simulink model "wlan_phy_rx_pmd"  (Simulink 7.8)
settings: Solver=VariableStepDiscrete  SolverMode=SingleTasking  StartTime=0.0  StopTime=rx_sim_time  RelTol=1e-3
PostLoadFcn:
  | wlan_phy_rx_init
InitFcn:
  | wlan_phy_rx_init
StartFcn:
  | wlan_phy_rx_init
values from neighbouring files (symbols this model uses):
  barker_seq20 = 0.95 * circshift(barker_seq20, [0 4]) ./ max(abs(barker_seq20))    [wlan_phy_rx_init.m]
  bit_scrambler_lfsr_bytes = sum(bit_scrambler_lfsr_bytes .* repmat(2.^[0:7], 127, 1),2)    [wlan_phy_rx_init.m]
  deinterleave_ROM = [deinterleave_ROM interleave_11n_64QAM zeros(1, 512-length(interleave_11n_64QAM))]    [wlan_phy_rx_init.m]
  longCorr_coef_i = [3*real(longCorr_coef)]    [wlan_phy_rx_init.m]
  longCorr_coef_q = [3*imag(longCorr_coef)]    [wlan_phy_rx_init.m]
  MAX_CP_LEN = 32    [wlan_phy_rx_init.m]
  MAX_NCBPS = NCBPS_11n_64QAM    (= 312)    [wlan_phy_rx_init.m]
  MAX_NUM_BYTES = 2^16-1    (= 65535)    [wlan_phy_rx_init.m]
  MAX_NUM_SC = 64    [wlan_phy_rx_init.m]
  MAX_NUM_SYMS = 600    [wlan_phy_rx_init.m]
  NCBPS_11a_BPSK = 48    [wlan_phy_rx_init.m]
  NDBPS_11a_BPSK12 = 24    [wlan_phy_rx_init.m]
  PHY_SIGNAL_MIN_LEN = 14    [wlan_phy_rx_init.m]
  preFFT_sampBuff_numSamps = 4*MAX_NUM_SC    (= 256)    [wlan_phy_rx_init.m]
  rx_sim_sig_samp_time = 8    [wlan_phy_rx_init.m]
  scr_ind_rev = zeros(1,128)    [wlan_phy_rx_init.m]
undefined symbols (not defined in the file or in its folder): gcbh, mcs_rom_11ag, mcs_rom_11n, xlBlockLoadCallback, xlGetNormalizedPeriod, xlipgetpartsetting
libraries: xbsIndex_r4 x3862, simulink x1, xbsEDKLib_r4 x1
(part 13 of 23)

== system wlan_phy_rx_pmd/DSSS Rx/Demod/Sync/SFD Search ==
  Bit : Inport
  Bit Valid : Inport  Port=2
  8 Shift Reg : Subsystem
  8 Shift Reg1 : Subsystem
  Concat1 : xbsIndex_r4/Concat  num_inputs=2  dbl_ovrd=off  block_type=concat
  Constant2 : xbsIndex_r4/Constant  const=hex2dec('F3A0')  gui_display_data_type=Fixed-point  arith_type=Unsigned  n_bits=16  bin_pt=0  preci_type=Single  exp_width=8  frac_width=24  explicit_period=off  period=1  equ=P=C  opselect=C  inp2=PCIN>>17  opr=+  inp1=P  carry=CIN  dbl_ovrd=off  block_type=constant
  Logical : xbsIndex_r4/Logical  logical_function=AND  inputs=2  en=off  latency=0  precision=Full  arith_type=Unsigned  n_bits=8  bin_pt=2  align_bp=on  dbl_ovrd=off  block_type=logical
      settings: xl_area=[0, 0, 0, 0, 0, 0, 0]  block_version=VER_STRING_GOES_HERE
  Posedge : Subsystem
  Relational1 : xbsIndex_r4/Relational  mode=a=b  en=off  latency=0  dbl_ovrd=off  block_type=relational
  Found : Outport
  wires:
  Logical/1 -> Posedge/1(D)
  Bit/1 -> 8 Shift Reg/1(D)
  Bit Valid/1 -> 8 Shift Reg/2(En), Logical/2, 8 Shift Reg1/2(En)
  [DSSS_RX_RESET] -> 8 Shift Reg/3(Rst), 8 Shift Reg1/3(Rst)
  8 Shift Reg/1(Q) -> 8 Shift Reg1/1(D)
  8 Shift Reg/2(8b) -> Concat1/1
  8 Shift Reg1/2(8b) -> Concat1/2
  Concat1/1 -> Relational1/2
  Constant2/1 -> Relational1/1
  Relational1/1 -> Logical/1
  Posedge/1(Q) -> Found/1

== system wlan_phy_rx_pmd/DSSS Rx/Sym Sync/Sym Sync Timeout/Posedge ==
  D : Inport
  Delay : xbsIndex_r4/Delay  rst=off  infoeditControl=Selection of Reset will increase slice count due to use of real FFs and instead of SRLs  en=off  latency=1  dbl_ovrd=off  reg_retiming=off  block_type=delay
      settings: infoedit=Hardware notes: A delay line is a chain, each link of which is an SRL16 followed by a flip-flop. If register retiming is enabled, the delay line is a chain of flip-flops.  xl_area=[1 1 0 0 0 0 0]  block_version=VER_STRING_GOES_HERE
  Inverter : xbsIndex_r4/Inverter  en=off  latency=0  dbl_ovrd=off  block_type=inv
      settings: xl_area=[0 0 0 0 0 0 0]  block_version=VER_STRING_GOES_HERE
  Logical1 : xbsIndex_r4/Logical  logical_function=AND  inputs=2  en=off  latency=0  precision=Full  arith_type=Unsigned  n_bits=8  bin_pt=2  align_bp=on  dbl_ovrd=off  block_type=logical
      settings: xl_area=[1 0 0 1 0 0 0]  block_version=VER_STRING_GOES_HERE
  Q : Outport
  wires:
  D/1 -> Logical1/2, Delay/1
  Logical1/1 -> Q/1
  Inverter/1 -> Logical1/1
  Delay/1 -> Inverter/1

== system wlan_phy_rx_pmd/DSSS Rx/Sym Sync/Sym Sync Timeout/S-R Latch2 ==
  S : Inport
  R : Inport  Port=2
  Constant1 : xbsIndex_r4/Constant  const=1  gui_display_data_type=Boolean  arith_type=Boolean  n_bits=1  bin_pt=0  preci_type=Single  exp_width=8  frac_width=24  explicit_period=off  period=1  equ=P=C  opselect=C  inp2=PCIN>>17  opr=+  inp1=P  carry=CIN  dbl_ovrd=off  block_type=constant
      settings: block_version=VER_STRING_GOES_HERE
  Convert1 : xbsIndex_r4/Convert  gui_display_data_type=Boolean  arith_type=Boolean  n_bits=1  bin_pt=0  float_type=Single  exp_bits=8  fraction_bits=24  quantization=Truncate  overflow=Wrap  en=off  latency=0  dbl_ovrd=off  pipeline=off  block_type=convert
  Convert2 : xbsIndex_r4/Convert  gui_display_data_type=Boolean  arith_type=Boolean  n_bits=1  bin_pt=0  float_type=Single  exp_bits=8  fraction_bits=24  quantization=Truncate  overflow=Wrap  en=off  latency=0  dbl_ovrd=off  pipeline=off  block_type=convert
  Register2 : xbsIndex_r4/Register  init=0  rst=on  en=on  dbl_ovrd=off  block_type=register
      settings: xl_area=[1 1 0 0 0 0 0]  block_version=VER_STRING_GOES_HERE
  Q : Outport
  wires:
  Convert2/1 -> Register2/3
  Convert1/1 -> Register2/2
  Constant1/1 -> Register2/1
  R/1 -> Convert1/1
  Register2/1 -> Q/1
  S/1 -> Convert2/1

== system wlan_phy_rx_pmd/Detect & Decode/De-Interleave & De-Puncture/Read Addr Gen/Ctrl ==
  note: This accumulator counts the number of actual bits that have been read
      | from the de-interleaver RAM. For 1/2 rate code this is easy (2 bits per read).
      | For the punctured code rates some read cycles represent 0, 1 or 2 bits. This is
      | important as the de-interleaver RAM only contains received bits and not the
      | zero-confidence values for de-punctured bits. De-puncturing is achieved by
      | not incrementing the read address and forcing the corresponding LLR
      | output to be zero (the value which means 'coin flip' for the decoder)
  mod_sel : Inport
  rate_sel : Inport  Port=2
  Reset : Inport  Port=3
  En : Inport  Port=4
  SIGNAL sym : Inport  Port=5
  Bit Index Calc : Subsystem
  Data Bits Count : Subsystem
  De Int ctrl : Scope  NumInputPorts=8
      settings: TimeRange=18000  YMin=0~0~0~0~0~0~-1~0  YMax=1~2~1~1~100~800~1~1  SaveName=ScopeData38
  Gateway Out{1..8} (8 alike) : xbsIndex_r4/Gateway Out  inherit_from_input=off  hdl_port=off  timing_constraint=None  locs_specified=off  LOCs={}  UseAsDAC=off  DACChannel='1'  block_type=gatewayout
  Mux1 : xbsIndex_r4/Mux  inputs=4  en=off  latency=0  precision=Full  arith_type=Unsigned  n_bits=16  bin_pt=14  quantization=Truncate  overflow=Wrap  dbl_ovrd=off  block_type=mux
  Mux3 : xbsIndex_r4/Mux  inputs=4  en=off  latency=0  precision=Full  arith_type=Unsigned  n_bits=16  bin_pt=14  quantization=Truncate  overflow=Wrap  dbl_ovrd=off  block_type=mux
  Mux4 : xbsIndex_r4/Mux  inputs=4  en=off  latency=0  precision=Full  arith_type=Unsigned  n_bits=16  bin_pt=14  quantization=Truncate  overflow=Wrap  dbl_ovrd=off  block_type=mux
  Rate 1/2 : Subsystem
  Rate 2/3 : Subsystem
  Rate 3/4 : Subsystem
  Rate 5/6 : Subsystem
  Ind A : Outport
  Ind B : Outport  Port=2
  Skip A : Outport  Port=3
  Skip B : Outport  Port=4
  Vout : Outport  Port=5
  Done : Outport  Port=6
  wires:
  Mux3/1 -> Skip A/1
  Rate 1/2/1(Ind Inc) -> [R12_INC_A]
  Rate 1/2/2(Skip A) -> [R12_SKIP_A]
  Rate 1/2/3(Skip B) -> [R12_SKIP_B]
  Rate 2/3/1(Ind Inc) -> [R23_INC_A]
  Rate 2/3/2(Skip A) -> [R23_SKIP_A]
  Rate 2/3/3(Skip B) -> [R23_SKIP_B]
  Rate 3/4/1(Ind Inc) -> [R34_INC_A]
  Rate 3/4/2(Skip A) -> [R34_SKIP_A]
  Rate 3/4/3(Skip B) -> [R34_SKIP_B]
  [R12_SKIP_A] -> Mux3/2
  [R23_SKIP_A] -> Mux3/3
  [R34_SKIP_A] -> Mux3/4
  Mux1/1 -> Skip B/1
  [R12_SKIP_B] -> Mux1/2
  [R23_SKIP_B] -> Mux1/3
  [R34_SKIP_B] -> Mux1/4
  [R12_INC_A] -> Mux4/2
  [R23_INC_A] -> Mux4/3
  [R34_INC_A] -> Mux4/4
  Mux4/1 -> Bit Index Calc/3(Inc)
  Gateway Out2/1 -> De Int ctrl/1  "Mod"
  Gateway Out1/1 -> De Int ctrl/2  "Rate"
  Gateway Out3/1 -> De Int ctrl/3  "Rst"
  Gateway Out4/1 -> De Int ctrl/4  "En"
  Gateway Out5/1 -> De Int ctrl/5  "A"
  Gateway Out7/1 -> De Int ctrl/8  "Vout"
  Data Bits Count/1(Done) -> Done/1, Gateway Out8/1
  Gateway Out8/1 -> De Int ctrl/7  "Done"
  mod_sel/1 -> Gateway Out2/1, Bit Index Calc/1(Mod)
  rate_sel/1 -> Mux4/1, Mux3/1, Mux1/1, Gateway Out1/1
  Reset/1 -> Gateway Out3/1, Bit Index Calc/4(Rst), Rate 2/3/1(Rst), Rate 3/4/1(Rst), Rate 5/6/1(Rst), Rate 1/2/1(Rst)
  En/1 -> Vout/1, Gateway Out7/1, Bit Index Calc/5(En), Rate 2/3/2(En), Rate 3/4/2(En), Rate 5/6/2(En), Rate 1/2/2(En), Gateway Out4/1, Data Bits Count/1(En)
  SIGNAL sym/1 -> Data Bits Count/2(SIGNAL sym), Bit Index Calc/2(SIGNAL Sym)
  Bit Index Calc/1(Ind A) -> Ind A/1, Gateway Out5/1
  Bit Index Calc/2(Ind B) -> Ind B/1, Gateway Out6/1  "B"
  Gateway Out6/1 -> De Int ctrl/6  "B"
  [R56_INC_A] -> Mux4/5
  [R56_SKIP_A] -> Mux3/5
  Rate 5/6/1(Ind Inc) -> [R56_INC_A]
  Rate 5/6/2(Skip A) -> [R56_SKIP_A]
  Rate 5/6/3(Skip B) -> [R56_SKIP_B]
  [R56_SKIP_B] -> Mux1/5

== system wlan_phy_rx_pmd/Detect & Decode/De-Interleave & De-Puncture/Read Addr Gen/De-Interleave DP-ROM ==
  Ind A : Inport
  Ind B : Inport  Port=2
  Constant8 : xbsIndex_r4/Constant  const=0  gui_display_data_type=Fixed-point  arith_type=Unsigned  n_bits=ceil(log2(MAX_NCBPS))  bin_pt=0  preci_type=Single  exp_width=8  frac_width=24  explicit_period=off  period=1  equ=P=C  opselect=C  inp2=PCIN>>17  opr=+  inp1=P  carry=CIN  dbl_ovrd=off  block_type=constant
  Constant9 : xbsIndex_r4/Constant  const=0  gui_display_data_type=Boolean  arith_type=Boolean  n_bits=2  bin_pt=0  preci_type=Single  exp_width=8  frac_width=24  explicit_period=off  period=1  equ=P=C  opselect=C  inp2=PCIN>>17  opr=+  inp1=P  carry=CIN  dbl_ovrd=off  block_type=constant
  De-Interleave ROM : xbsIndex_r4/Dual Port RAM  depth=length(deinterleave_ROM)  initVector=deinterleave_ROM  distributed_mem=Block RAM  init_a=0  init_b=0  rst_a=off  rst_b=off  en_a=off  en_b=off  latency=1  write_mode_A=Read After Write  write_mode_B=Read After Write  dbl_ovrd=off  optimize=Area  block_type=dpram
  Addr A : Outport
  Addr B : Outport  Port=2
  wires:
  Constant8/1 -> De-Interleave ROM/2, De-Interleave ROM/5
  Constant9/1 -> De-Interleave ROM/3, De-Interleave ROM/6
  Ind A/1 -> De-Interleave ROM/1
  De-Interleave ROM/1 -> Addr A/1
  Ind B/1 -> De-Interleave ROM/4
  De-Interleave ROM/2 -> Addr B/1

== system wlan_phy_rx_pmd/Detect & Decode/De-Interleave & De-Puncture/Read Addr Gen/S-R Latch1 ==
  S : Inport
  R : Inport  Port=2
  Constant1 : xbsIndex_r4/Constant  const=1  gui_display_data_type=Fixed-point  arith_type=Boolean  n_bits=1  bin_pt=0  preci_type=Single  exp_width=8  frac_width=24  explicit_period=off  period=1  equ=P=C  opselect=C  inp2=PCIN>>17  opr=+  inp1=P  carry=CIN  dbl_ovrd=off  block_type=constant
      settings: block_version=VER_STRING_GOES_HERE
  Convert1 : xbsIndex_r4/Convert  gui_display_data_type=Boolean  arith_type=Boolean  n_bits=1  bin_pt=0  float_type=Single  exp_bits=8  fraction_bits=24  quantization=Truncate  overflow=Wrap  en=off  latency=0  dbl_ovrd=off  pipeline=off  block_type=convert
  Convert2 : xbsIndex_r4/Convert  gui_display_data_type=Boolean  arith_type=Boolean  n_bits=1  bin_pt=0  float_type=Single  exp_bits=8  fraction_bits=24  quantization=Truncate  overflow=Wrap  en=off  latency=0  dbl_ovrd=off  pipeline=off  block_type=convert
  Register2 : xbsIndex_r4/Register  init=0  rst=on  en=on  dbl_ovrd=off  block_type=register
      settings: xl_area=[1 1 0 0 0 0 0]  block_version=VER_STRING_GOES_HERE
  Q : Outport
  wires:
  Convert2/1 -> Register2/3
  Convert1/1 -> Register2/2
  Constant1/1 -> Register2/1
  R/1 -> Convert1/1
  Register2/1 -> Q/1
  S/1 -> Convert2/1

== system wlan_phy_rx_pmd/Detect & Decode/Decode/Byte Count/S-R Latch1 ==
  S : Inport
  R : Inport  Port=2
  Constant1 : xbsIndex_r4/Constant  const=1  gui_display_data_type=Fixed-point  arith_type=Boolean  n_bits=1  bin_pt=0  preci_type=Single  exp_width=8  frac_width=24  explicit_period=off  period=1  equ=P=C  opselect=C  inp2=PCIN>>17  opr=+  inp1=P  carry=CIN  dbl_ovrd=off  block_type=constant
      settings: block_version=VER_STRING_GOES_HERE
  Convert1 : xbsIndex_r4/Convert  gui_display_data_type=Boolean  arith_type=Boolean  n_bits=1  bin_pt=0  float_type=Single  exp_bits=8  fraction_bits=24  quantization=Truncate  overflow=Wrap  en=off  latency=0  dbl_ovrd=off  pipeline=off  block_type=convert
  Convert2 : xbsIndex_r4/Convert  gui_display_data_type=Boolean  arith_type=Boolean  n_bits=1  bin_pt=0  float_type=Single  exp_bits=8  fraction_bits=24  quantization=Truncate  overflow=Wrap  en=off  latency=0  dbl_ovrd=off  pipeline=off  block_type=convert
  Register2 : xbsIndex_r4/Register  init=0  rst=on  en=on  dbl_ovrd=off  block_type=register
      settings: xl_area=[1 1 0 0 0 0 0]  block_version=VER_STRING_GOES_HERE
  Q : Outport
  wires:
  Convert2/1 -> Register2/3
  Convert1/1 -> Register2/2
  Constant1/1 -> Register2/1
  R/1 -> Convert1/1
  Register2/1 -> Q/1
  S/1 -> Convert2/1

== system wlan_phy_rx_pmd/Detect & Decode/Decode/Control/Early Trace Ctrl ==
  note: 22: (NUM_SIGNAL_BYTES * 8) - 2
  note: 70: ((NUM_SIGNAL_BYTES + NUM_HT_SIGNAL_BYTES)* 8) - 2
  note: early_trace inputs require pulse coincident
      | with last vin for early bits, staying asserted
      | until at least first decoder vout.
  Byte Ind : Inport
  dec_vout : Inport  Port=2
  reset : Inport  Port=3
  Constant1 : xbsIndex_r4/Constant  const=9*8 - 2  gui_display_data_type=Fixed-point  arith_type=Unsigned  n_bits=7  bin_pt=0  preci_type=Single  exp_width=8  frac_width=24  explicit_period=off  period=1  equ=P=C  opselect=C  inp2=PCIN>>17  opr=+  inp1=P  carry=CIN  dbl_ovrd=off  block_type=constant
  Constant3 : xbsIndex_r4/Constant  const=3*8 - 2  gui_display_data_type=Fixed-point  arith_type=Unsigned  n_bits=5  bin_pt=0  preci_type=Single  exp_width=8  frac_width=24  explicit_period=off  period=1  equ=P=C  opselect=C  inp2=PCIN>>17  opr=+  inp1=P  carry=CIN  dbl_ovrd=off  block_type=constant
  Convert : xbsIndex_r4/Convert  gui_display_data_type=Boolean  arith_type=Boolean  n_bits=16  bin_pt=14  float_type=Single  exp_bits=8  fraction_bits=24  quantization=Truncate  overflow=Wrap  en=off  latency=0  dbl_ovrd=off  pipeline=off  block_type=convert
  Convert1 : xbsIndex_r4/Convert  gui_display_data_type=Boolean  arith_type=Boolean  n_bits=16  bin_pt=14  float_type=Single  exp_bits=8  fraction_bits=24  quantization=Truncate  overflow=Wrap  en=off  latency=0  dbl_ovrd=off  pipeline=off  block_type=convert
  Logical1 : xbsIndex_r4/Logical  logical_function=AND  inputs=2  en=off  latency=0  precision=Full  arith_type=Unsigned  n_bits=16  bin_pt=0  align_bp=on  dbl_ovrd=off  block_type=logical
  Logical2 : xbsIndex_r4/Logical  logical_function=OR  inputs=2  en=off  latency=0  precision=Full  arith_type=Unsigned  n_bits=16  bin_pt=0  align_bp=on  dbl_ovrd=off  block_type=logical
  Relational1 : xbsIndex_r4/Relational  mode=a=b  en=off  latency=0  dbl_ovrd=off  block_type=relational
  Relational3 : xbsIndex_r4/Relational  mode=a=b  en=off  latency=0  dbl_ovrd=off  block_type=relational
  S-R Latch1 : Subsystem
  S-R Latch3 : Subsystem
  posedge : Subsystem
  posedge1 : Subsystem
  Early Trace 1 : Outport
  Early Trace 2 : Outport  Port=2
  wires:
  Constant3/1 -> Relational3/1
  Byte Ind/1 -> Relational3/2, Relational1/1
  Relational3/1 -> posedge/1(D)
  Constant1/1 -> Relational1/2
  Relational1/1 -> Logical1/1
  [RX_PHY_MODE_11N_AC] -> Logical1/2
  Logical1/1 -> posedge1/1(D)
  posedge/1(Q) -> S-R Latch1/1(S)
  posedge1/1(Q) -> S-R Latch3/1(S)
  dec_vout/1 -> Convert/1
  reset/1 -> Convert1/1
  Logical2/1 -> S-R Latch1/2(R), S-R Latch3/2(R)
  S-R Latch1/1(Q) -> Early Trace 1/1
  S-R Latch3/1(Q) -> Early Trace 2/1
  Convert/1 -> Logical2/1
  Convert1/1 -> Logical2/2

== system wlan_phy_rx_pmd/Detect & Decode/Decode/Control/negedge ==
  D : Inport
  Delay : xbsIndex_r4/Delay  rst=off  infoeditControl=Selection of Reset will increase slice count due to use of real FFs and instead of SRLs  en=off  latency=1  dbl_ovrd=off  reg_retiming=off  block_type=delay
      settings: infoedit=Hardware notes: A delay line is a chain, each link of which is an SRL16 followed by a flip-flop. If register retiming is enabled, the delay line is a chain of flip-flops.  xl_area=[1 1 0 0 0 0 0]  block_version=VER_STRING_GOES_HERE
  Inverter : xbsIndex_r4/Inverter  en=off  latency=0  dbl_ovrd=off  block_type=inv
      settings: xl_area=[0 0 0 0 0 0 0]  block_version=VER_STRING_GOES_HERE
  Logical1 : xbsIndex_r4/Logical  logical_function=AND  inputs=2  en=off  latency=0  precision=Full  arith_type=Unsigned  n_bits=8  bin_pt=2  align_bp=on  dbl_ovrd=off  block_type=logical
      settings: xl_area=[1 0 0 1 0 0 0]  block_version=VER_STRING_GOES_HERE
  Q : Outport
  wires:
  D/1 -> Delay/1, Inverter/1
  Logical1/1 -> Q/1
  Delay/1 -> Logical1/1
  Inverter/1 -> Logical1/2

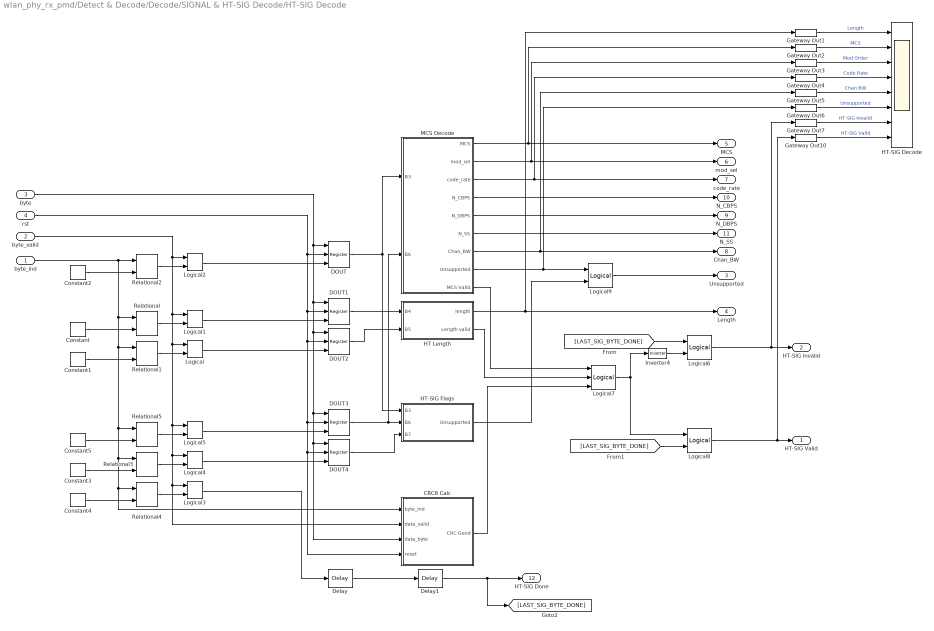
== system wlan_phy_rx_pmd/Detect & Decode/Decode/SIGNAL & HT-SIG Decode/HT-SIG Decode ==
  byte_ind : Inport
  byte_valid : Inport  Port=2
  byte : Inport  Port=3
  rst : Inport  Port=4
  CRC8 Calc : Subsystem
  Constant : xbsIndex_r4/Constant  const=4  gui_display_data_type=Fixed-point  arith_type=Unsigned  n_bits=3  bin_pt=0  preci_type=Single  exp_width=8  frac_width=24  explicit_period=off  period=1  equ=P=C  opselect=C  inp2=PCIN>>17  opr=+  inp1=P  carry=CIN  dbl_ovrd=off  block_type=constant
  Constant1 : xbsIndex_r4/Constant  const=5  gui_display_data_type=Fixed-point  arith_type=Unsigned  n_bits=3  bin_pt=0  preci_type=Single  exp_width=8  frac_width=24  explicit_period=off  period=1  equ=P=C  opselect=C  inp2=PCIN>>17  opr=+  inp1=P  carry=CIN  dbl_ovrd=off  block_type=constant
  Constant2 : xbsIndex_r4/Constant  const=3  gui_display_data_type=Fixed-point  arith_type=Unsigned  n_bits=2  bin_pt=0  preci_type=Single  exp_width=8  frac_width=24  explicit_period=off  period=1  equ=P=C  opselect=C  inp2=PCIN>>17  opr=+  inp1=P  carry=CIN  dbl_ovrd=off  block_type=constant
  Constant3 : xbsIndex_r4/Constant  const=7  gui_display_data_type=Fixed-point  arith_type=Unsigned  n_bits=3  bin_pt=0  preci_type=Single  exp_width=8  frac_width=24  explicit_period=off  period=1  equ=P=C  opselect=C  inp2=PCIN>>17  opr=+  inp1=P  carry=CIN  dbl_ovrd=off  block_type=constant
  Constant4 : xbsIndex_r4/Constant  const=8  gui_display_data_type=Fixed-point  arith_type=Unsigned  n_bits=4  bin_pt=0  preci_type=Single  exp_width=8  frac_width=24  explicit_period=off  period=1  equ=P=C  opselect=C  inp2=PCIN>>17  opr=+  inp1=P  carry=CIN  dbl_ovrd=off  block_type=constant
  Constant5 : xbsIndex_r4/Constant  const=6  gui_display_data_type=Fixed-point  arith_type=Unsigned  n_bits=3  bin_pt=0  preci_type=Single  exp_width=8  frac_width=24  explicit_period=off  period=1  equ=P=C  opselect=C  inp2=PCIN>>17  opr=+  inp1=P  carry=CIN  dbl_ovrd=off  block_type=constant
  DOUT : xbsIndex_r4/Register  init=0  rst=on  en=on  dbl_ovrd=off  block_type=register
  DOUT1 : xbsIndex_r4/Register  init=100  rst=on  en=on  dbl_ovrd=off  block_type=register
  DOUT2 : xbsIndex_r4/Register  init=0  rst=on  en=on  dbl_ovrd=off  block_type=register
  DOUT3 : xbsIndex_r4/Register  init=hex2dec('03')  rst=on  en=on  dbl_ovrd=off  block_type=register
  DOUT4 : xbsIndex_r4/Register  init=hex2dec('E0')  rst=on  en=on  dbl_ovrd=off  block_type=register
  Delay : xbsIndex_r4/Delay  rst=off  infoeditControl=Selection of Reset will increase slice count due to use of real FFs and instead of SRLs  en=off  latency=1  dbl_ovrd=off  reg_retiming=off  block_type=delay
  Delay1 : xbsIndex_r4/Delay  rst=off  infoeditControl=Selection of Reset will increase slice count due to use of real FFs and instead of SRLs  en=off  latency=1  dbl_ovrd=off  reg_retiming=off  block_type=delay
  Gateway Out{1..7,10} (8 alike) : xbsIndex_r4/Gateway Out  inherit_from_input=off  hdl_port=off  timing_constraint=None  locs_specified=off  LOCs={}  UseAsDAC=off  DACChannel='1'  block_type=gatewayout
  HT Length : Subsystem
  HT-SIG Decode : Scope  NumInputPorts=8
      settings: TimeRange=7000  YMin=0~0~0~0~100~-1~-1~-1  YMax=1~1~150~1~300~1~1~1  SaveName=ScopeData45
  HT-SIG Flags : Subsystem
  Inverter4 : xbsIndex_r4/Inverter  en=off  latency=0  dbl_ovrd=off  block_type=inv
  Logical{,1..6,8} (8 alike) : xbsIndex_r4/Logical  logical_function=AND  inputs=2  en=off  latency=0  precision=Full  arith_type=Unsigned  n_bits=16  bin_pt=0  align_bp=on  dbl_ovrd=off  block_type=logical
  Logical7 : xbsIndex_r4/Logical  logical_function=AND  inputs=3  en=off  latency=0  precision=Full  arith_type=Unsigned  n_bits=16  bin_pt=0  align_bp=on  dbl_ovrd=off  block_type=logical
  Logical9 : xbsIndex_r4/Logical  logical_function=OR  inputs=2  en=off  latency=0  precision=Full  arith_type=Unsigned  n_bits=16  bin_pt=0  align_bp=on  dbl_ovrd=off  block_type=logical
  MCS Decode : Subsystem
  Relational{,1..5} (6 alike) : xbsIndex_r4/Relational  mode=a=b  en=off  latency=0  dbl_ovrd=off  block_type=relational
  HT-SIG Valid : Outport
  HT-SIG Invalid : Outport  Port=2
  Unsupported : Outport  Port=3
  Length : Outport  Port=4
  MCS : Outport  Port=5
  mod_sel : Outport  Port=6
  code_rate : Outport  Port=7
  Chan_BW : Outport  Port=8
  N_DBPS : Outport  Port=9
  N_CBPS : Outport  Port=10
  N_SS : Outport  Port=11
  HT-SIG Done : Outport  Port=12
  wires:
  rst/1 -> DOUT1/2, DOUT2/2, DOUT3/2, DOUT4/2, CRC8 Calc/4(reset), DOUT/2
  Logical1/1 -> DOUT1/3
  Logical2/1 -> DOUT/3
  Logical/1 -> DOUT2/3
  Relational/1 -> Logical1/2
  Relational2/1 -> Logical2/2
  Relational1/1 -> Logical/2
  Constant2/1 -> Relational2/2
  Constant1/1 -> Relational1/2
  Constant/1 -> Relational/2
  byte/1 -> DOUT1/1, DOUT2/1, DOUT4/1, CRC8 Calc/3(data_byte), DOUT3/1, DOUT/1
  byte_ind/1 -> Relational/1, Relational1/1, Relational3/1, Relational4/1, CRC8 Calc/1(byte_ind), Relational5/1, Relational2/1
  byte_valid/1 -> Logical1/1, Logical/1, Logical4/1, Logical3/1, CRC8 Calc/2(data_valid), Logical5/1, Logical2/1
  Logical4/1 -> DOUT4/3
  Logical5/1 -> DOUT3/3
  Logical3/1 -> Delay/1
  Relational3/1 -> Logical4/2
  Relational5/1 -> Logical5/2
  Relational4/1 -> Logical3/2
  Constant5/1 -> Relational5/2
  Constant4/1 -> Relational4/2
  Constant3/1 -> Relational3/2
  DOUT/1 -> HT-SIG Flags/1(B3), MCS Decode/1(B3)
  DOUT1/1 -> HT Length/1(B4)
  DOUT2/1 -> HT Length/2(B5)
  DOUT3/1 -> HT-SIG Flags/2(B6), MCS Decode/2(B6)
  Gateway Out1/1 -> HT-SIG Decode/1  "Length"
  Gateway Out10/1 -> HT-SIG Decode/8  "HT-SIG Valid"
  Gateway Out2/1 -> HT-SIG Decode/2  "MCS"
  Gateway Out3/1 -> HT-SIG Decode/3  "Mod Order"
  Gateway Out4/1 -> HT-SIG Decode/4  "Code Rate"
  MCS Decode/1(MCS) -> MCS/1, Gateway Out2/1
  MCS Decode/2(mod_sel) -> mod_sel/1, Gateway Out3/1
  MCS Decode/3(code_rate) -> code_rate/1, Gateway Out4/1
  MCS Decode/4(N_CBPS) -> N_CBPS/1
  MCS Decode/5(N_DBPS) -> N_DBPS/1
  MCS Decode/6(N_SS) -> N_SS/1
  MCS Decode/7(Chan_BW) -> Chan_BW/1, Gateway Out5/1
  HT Length/1(length) -> Length/1, Gateway Out1/1
  [LAST_SIG_BYTE_DONE] -> Logical6/1
  Logical7/1 -> Inverter4/1, Logical8/1
  [LAST_SIG_BYTE_DONE] -> Logical8/2
  Logical6/1 -> HT-SIG Invalid/1, Gateway Out7/1
  Logical8/1 -> HT-SIG Valid/1, Gateway Out10/1
  MCS Decode/9(MCS Valid) -> Logical7/1
  HT Length/2(Length valid) -> Logical7/2
  CRC8 Calc/1(CRC Good) -> Logical7/3
  Delay/1 -> Delay1/1
  HT-SIG Flags/1(Unsupported) -> Logical9/2
  MCS Decode/8(Unsupported) -> Gateway Out6/1, Logical9/1
  Gateway Out5/1 -> HT-SIG Decode/5  "Chan BW"
  Gateway Out6/1 -> HT-SIG Decode/6  "Unsupported"
  Delay1/1 -> [LAST_SIG_BYTE_DONE], HT-SIG Done/1
  DOUT4/1 -> HT-SIG Flags/3(B7)
  Logical9/1 -> Unsupported/1
  Gateway Out7/1 -> HT-SIG Decode/7  "HT-SIG Invalid"
  Inverter4/1 -> Logical6/2

== system wlan_phy_rx_pmd/Detect & Decode/Decode/SIGNAL & HT-SIG Decode/S-R Latch1 ==
  S : Inport
  R : Inport  Port=2
  Constant1 : xbsIndex_r4/Constant  const=1  gui_display_data_type=Fixed-point  arith_type=Boolean  n_bits=1  bin_pt=0  preci_type=Single  exp_width=8  frac_width=24  explicit_period=off  period=1  equ=P=C  opselect=C  inp2=PCIN>>17  opr=+  inp1=P  carry=CIN  dbl_ovrd=off  block_type=constant
      settings: block_version=VER_STRING_GOES_HERE
  Convert1 : xbsIndex_r4/Convert  gui_display_data_type=Boolean  arith_type=Boolean  n_bits=1  bin_pt=0  float_type=Single  exp_bits=8  fraction_bits=24  quantization=Truncate  overflow=Wrap  en=off  latency=0  dbl_ovrd=off  pipeline=off  block_type=convert
  Convert2 : xbsIndex_r4/Convert  gui_display_data_type=Boolean  arith_type=Boolean  n_bits=1  bin_pt=0  float_type=Single  exp_bits=8  fraction_bits=24  quantization=Truncate  overflow=Wrap  en=off  latency=0  dbl_ovrd=off  pipeline=off  block_type=convert
  Register2 : xbsIndex_r4/Register  init=0  rst=on  en=on  dbl_ovrd=off  block_type=register
      settings: xl_area=[1 1 0 0 0 0 0]  block_version=VER_STRING_GOES_HERE
  Q : Outport
  wires:
  Convert2/1 -> Register2/3
  Convert1/1 -> Register2/2
  Constant1/1 -> Register2/1
  R/1 -> Convert1/1
  Register2/1 -> Q/1
  S/1 -> Convert2/1

== system wlan_phy_rx_pmd/Detect & Decode/Decode/SIGNAL & HT-SIG Decode/SIGNAL Decode ==
  note: Init values of these regs are important
      | Decoder black box requires valid params at start,
      | so these regs are initialzied such that:
      | mod_order = 0 (BSPK)
      | code_rate = 0 (1/2)
      | num_bytes = 64 (sane default)
      |
      | The choice of BPSK with 1/2 code rate is critical to
      | allow demodulation of the SIGNAL field (first OFDM
      | symbol) and potential HT/VHT-SIG fields (next 3
      | OFDM symbols).
  byte_ind : Inport
  byte_valid : Inport  Port=2
  byte : Inport  Port=3
  rst : Inport  Port=4
  4b Shift Reg : Subsystem
  Constant : xbsIndex_r4/Constant  const=1  gui_display_data_type=Fixed-point  arith_type=Unsigned  n_bits=2  bin_pt=0  preci_type=Single  exp_width=8  frac_width=24  explicit_period=off  period=1  equ=P=C  opselect=C  inp2=PCIN>>17  opr=+  inp1=P  carry=CIN  dbl_ovrd=off  block_type=constant
  Constant1 : xbsIndex_r4/Constant  const=2  gui_display_data_type=Fixed-point  arith_type=Unsigned  n_bits=2  bin_pt=0  preci_type=Single  exp_width=8  frac_width=24  explicit_period=off  period=1  equ=P=C  opselect=C  inp2=PCIN>>17  opr=+  inp1=P  carry=CIN  dbl_ovrd=off  block_type=constant
  Constant2 : xbsIndex_r4/Constant  const=0  gui_display_data_type=Fixed-point  arith_type=Unsigned  n_bits=2  bin_pt=0  preci_type=Single  exp_width=8  frac_width=24  explicit_period=off  period=1  equ=P=C  opselect=C  inp2=PCIN>>17  opr=+  inp1=P  carry=CIN  dbl_ovrd=off  block_type=constant
  DOUT : xbsIndex_r4/Register  init=hex2dec('6B')  rst=on  en=on  dbl_ovrd=off  block_type=register
  DOUT1 : xbsIndex_r4/Register  init=hex2dec('07')  rst=on  en=on  dbl_ovrd=off  block_type=register
  DOUT2 : xbsIndex_r4/Register  init=hex2dec('00')  rst=on  en=on  dbl_ovrd=off  block_type=register
  DOUT6 : xbsIndex_r4/Register  init=0  rst=off  en=off  dbl_ovrd=off  block_type=register
  DOUT7 : xbsIndex_r4/Register  init=0  rst=off  en=off  dbl_ovrd=off  block_type=register
  Delay : xbsIndex_r4/Delay  rst=off  infoeditControl=Selection of Reset will increase slice count due to use of real FFs and instead of SRLs  en=off  latency=1  dbl_ovrd=off  reg_retiming=off  block_type=delay
  Inverter3 : xbsIndex_r4/Inverter  en=off  latency=0  dbl_ovrd=off  block_type=inv
  Inverter4 : xbsIndex_r4/Inverter  en=off  latency=0  dbl_ovrd=off  block_type=inv
  LENGTH : Subsystem
  Logical{,1..3,6} (5 alike) : xbsIndex_r4/Logical  logical_function=AND  inputs=2  en=off  latency=0  precision=Full  arith_type=Unsigned  n_bits=16  bin_pt=0  align_bp=on  dbl_ovrd=off  block_type=logical
  Logical5 : xbsIndex_r4/Logical  logical_function=OR  inputs=3  en=off  latency=0  precision=Full  arith_type=Unsigned  n_bits=16  bin_pt=0  align_bp=on  dbl_ovrd=off  block_type=logical
  RATE Decode : Subsystem
  Relational : xbsIndex_r4/Relational  mode=a=b  en=off  latency=0  dbl_ovrd=off  block_type=relational
  Relational1 : xbsIndex_r4/Relational  mode=a=b  en=off  latency=0  dbl_ovrd=off  block_type=relational
  Relational2 : xbsIndex_r4/Relational  mode=a=b  en=off  latency=0  dbl_ovrd=off  block_type=relational
  SIGNAL Dec : Scope  NumInputPorts=8
      settings: TimeRange=19620  YMin=50~0~-1~10~-1~-1~-5~-5  YMax=100~1~1~11~1~1~5~5  SaveName=ScopeData18
  Tail & Parity Check : Subsystem
  dbg_SIGNAL_ERR_DISP : xbsIndex_r4/Gateway Out  inherit_from_input=off  hdl_port=on  timing_constraint=None  locs_specified=off  LOCs={}  UseAsDAC=off  DACChannel='1'  block_type=gatewayout
  int{,1..7} (8 alike) : xbsIndex_r4/Gateway Out  inherit_from_input=off  hdl_port=off  timing_constraint=None  locs_specified=off  LOCs={}  UseAsDAC=off  DACChannel='1'  block_type=gatewayout
  SIGNAL Valid : Outport
  SIGNAL Invalid : Outport  Port=2
  Length : Outport  Port=3
  MCS : Outport  Port=4
  mod_sel : Outport  Port=5
  code_rate : Outport  Port=6
  Chan_BW : Outport  Port=7
  N_DBPS : Outport  Port=8
  N_CBPS : Outport  Port=9
  N_SS : Outport  Port=10
  SIGNAL Done : Outport  Port=11
  wires:
  4b Shift Reg/1(Q) -> DOUT6/1
  DOUT7/1 -> dbg_SIGNAL_ERR_DISP/1
  DOUT6/1 -> DOUT7/1
  DOUT2/1 -> Tail & Parity Check/3(B2), LENGTH/3(B2)
  DOUT1/1 -> Tail & Parity Check/2(B1), LENGTH/2(B1)
  DOUT/1 -> Tail & Parity Check/1(B0), LENGTH/1(B0), RATE Decode/1(B0)
  rst/1 -> DOUT/2, DOUT1/2, DOUT2/2
  Logical1/1 -> DOUT1/3
  Logical2/1 -> DOUT/3
  Logical/1 -> DOUT2/3, Delay/1
  Relational/1 -> Logical1/2
  Relational2/1 -> Logical2/2
  Relational1/1 -> Logical/2
  Constant2/1 -> Relational2/2
  Constant1/1 -> Relational1/2
  Constant/1 -> Relational/2
  byte/1 -> DOUT2/1, DOUT1/1, DOUT/1
  byte_ind/1 -> Relational/1, Relational1/1, Relational2/1
  byte_valid/1 -> Logical/1, Logical1/1, Logical2/1
  Delay/1 -> [LAST_SIG_BYTE_DONE], SIGNAL Done/1
  RATE Decode/1(MCS) -> MCS/1, int1/1
  RATE Decode/2(mod_sel) -> mod_sel/1, int2/1
  RATE Decode/3(code_rate) -> code_rate/1, int3/1
  RATE Decode/4(N_CBPS) -> N_CBPS/1
  RATE Decode/5(N_DBPS) -> N_DBPS/1, int7/1
  RATE Decode/6(N_SS) -> N_SS/1
  RATE Decode/7(Chan_BW) -> Chan_BW/1
  RATE Decode/8(RATE Valid) -> Inverter3/1
  [LAST_SIG_BYTE_DONE] -> Logical3/1
  Logical3/1 -> SIGNAL Invalid/1, 4b Shift Reg/1(En), int4/1
  Inverter3/1 -> Logical5/1
  LENGTH/1(len invalid) -> Logical5/2
  Tail & Parity Check/1(Invalid) -> Logical5/3
  Logical5/1 -> Logical3/2, Inverter4/1
  [LAST_SIG_BYTE_DONE] -> Logical6/2
  Inverter4/1 -> Logical6/1
  Logical6/1 -> SIGNAL Valid/1, int5/1
  LENGTH/2(Length) -> Length/1, int6/1  "Length"
  int/1 -> SIGNAL Dec/1  "SIG Done"
  [LAST_SIG_BYTE_DONE] -> int/1
  int2/1 -> SIGNAL Dec/3  "Mod order"
  int1/1 -> SIGNAL Dec/2  "MCS"
  int3/1 -> SIGNAL Dec/4  "Code rate"
  int4/1 -> SIGNAL Dec/6  "SIG Invalid"
  int5/1 -> SIGNAL Dec/7  "SIG Valid"
  int6/1 -> SIGNAL Dec/5  "Length"
  int7/1 -> SIGNAL Dec/8  "N_DBPS"

== system wlan_phy_rx_pmd/Detect & Decode/Decode/Safe ToUint8/Indet Check ==
  In1 : Inport
  Constant : xbsIndex_r4/Constant  const=0  gui_display_data_type=Fixed-point  arith_type=Unsigned  n_bits=8  bin_pt=0  preci_type=Single  exp_width=8  frac_width=24  explicit_period=off  period=1  equ=P=C  opselect=C  inp2=PCIN>>17  opr=+  inp1=P  carry=CIN  dbl_ovrd=off  block_type=constant
  Disregard Subsystem : xbsIndex_r4/Disregard Subsystem  Tag=discardX  block_type=disregard
  Gateway In : xbsIndex_r4/Gateway In  gui_display_data_type=Boolean  arith_type=Boolean  n_bits=16  bin_pt=14  preci_type=Single  exp_width=8  frac_width=24  quantization="Round  (unbiased: +/- Inf)"  overflow=Saturate  period=1  dbl_ovrd=off  timing_constraint=None  locs_specified=off  LOCs={}  UseAsADC=off  ADCChannel='1'  inherit_from_input=off  hdl_port=on  block_type=gatewayin
  Indeterminate Probe : xbsIndex_r4/Indeterminate Probe  block_type=indetprobe
      settings: infoedit=Produces an output 1 of type double when input data is indeterminate. Output is 0 otherwise.<br><br>Hardware notes: This block and any downstream blocks will be trimmed when hardware is generated.
  Mux1 : xbsIndex_r4/Mux  inputs=2  en=off  latency=0  precision=Full  arith_type=Unsigned  n_bits=16  bin_pt=14  quantization=Truncate  overflow=Wrap  dbl_ovrd=off  block_type=mux
  Out1 : Outport
  wires:
  Gateway In/1 -> Mux1/1
  Constant/1 -> Mux1/3
  Indeterminate Probe/1 -> Gateway In/1
  Mux1/1 -> Out1/1
  In1/1 -> Mux1/2, Indeterminate Probe/1

== system wlan_phy_rx_pmd/Detect & Decode/Decode/Stretch/S-R Latch1 ==
  S : Inport
  R : Inport  Port=2
  Constant1 : xbsIndex_r4/Constant  const=1  gui_display_data_type=Fixed-point  arith_type=Boolean  n_bits=1  bin_pt=0  preci_type=Single  exp_width=8  frac_width=24  explicit_period=off  period=1  equ=P=C  opselect=C  inp2=PCIN>>17  opr=+  inp1=P  carry=CIN  dbl_ovrd=off  block_type=constant
      settings: block_version=VER_STRING_GOES_HERE
  Convert1 : xbsIndex_r4/Convert  gui_display_data_type=Boolean  arith_type=Boolean  n_bits=1  bin_pt=0  float_type=Single  exp_bits=8  fraction_bits=24  quantization=Truncate  overflow=Wrap  en=off  latency=0  dbl_ovrd=off  pipeline=off  block_type=convert
  Convert2 : xbsIndex_r4/Convert  gui_display_data_type=Boolean  arith_type=Boolean  n_bits=1  bin_pt=0  float_type=Single  exp_bits=8  fraction_bits=24  quantization=Truncate  overflow=Wrap  en=off  latency=0  dbl_ovrd=off  pipeline=off  block_type=convert
  Register2 : xbsIndex_r4/Register  init=0  rst=on  en=on  dbl_ovrd=off  block_type=register
      settings: xl_area=[1 1 0 0 0 0 0]  block_version=VER_STRING_GOES_HERE
  Q : Outport
  wires:
  Constant1/1 -> Register2/1
  R/1 -> Convert1/1
  Register2/1 -> Q/1
  S/1 -> Convert2/1
  Convert1/1 -> Register2/2
  Convert2/1 -> Register2/3

== system wlan_phy_rx_pmd/Detect & Decode/Descramble/Descram Byte Calc/LFSR Init ==
  B : Inport
  7LSB : xbsIndex_r4/Slice  nbits=7  boolean_output=off  mode=Lower Bit Location + Width  bit1=0  base1=MSB of Input  bit0=0  base0=LSB of Input  dbl_ovrd=off  block_type=slice
  7MSB : xbsIndex_r4/Slice  nbits=7  boolean_output=off  mode=Upper Bit Location + Width  bit1=0  base1=MSB of Input  bit0=0  base0=LSB of Input  dbl_ovrd=off  block_type=slice
  Concat : xbsIndex_r4/Concat  num_inputs=7  dbl_ovrd=off  block_type=concat
  b0 : xbsIndex_r4/Slice  nbits=1  boolean_output=off  mode=Lower Bit Location + Width  bit1=0  base1=MSB of Input  bit0=0  base0=LSB of Input  dbl_ovrd=off  block_type=slice
  b1 : xbsIndex_r4/Slice  nbits=1  boolean_output=off  mode=Lower Bit Location + Width  bit1=0  base1=MSB of Input  bit0=1  base0=LSB of Input  dbl_ovrd=off  block_type=slice
  b2 : xbsIndex_r4/Slice  nbits=1  boolean_output=off  mode=Lower Bit Location + Width  bit1=0  base1=MSB of Input  bit0=2  base0=LSB of Input  dbl_ovrd=off  block_type=slice
  b3 : xbsIndex_r4/Slice  nbits=1  boolean_output=off  mode=Lower Bit Location + Width  bit1=0  base1=MSB of Input  bit0=3  base0=LSB of Input  dbl_ovrd=off  block_type=slice
  b4 : xbsIndex_r4/Slice  nbits=1  boolean_output=off  mode=Lower Bit Location + Width  bit1=0  base1=MSB of Input  bit0=4  base0=LSB of Input  dbl_ovrd=off  block_type=slice
  b5 : xbsIndex_r4/Slice  nbits=1  boolean_output=off  mode=Lower Bit Location + Width  bit1=0  base1=MSB of Input  bit0=5  base0=LSB of Input  dbl_ovrd=off  block_type=slice
  b6 : xbsIndex_r4/Slice  nbits=1  boolean_output=off  mode=Lower Bit Location + Width  bit1=0  base1=MSB of Input  bit0=6  base0=LSB of Input  dbl_ovrd=off  block_type=slice
  I : Outport
  wires:
  Concat/1 -> ?
  b6/1 -> Concat/7
  b5/1 -> Concat/6
  b4/1 -> Concat/5
  b3/1 -> Concat/4
  b2/1 -> Concat/3
  b1/1 -> Concat/2
  b0/1 -> Concat/1
  7MSB/1 -> b6/1, b5/1, b4/1, b3/1, b2/1, b1/1, b0/1
  B/1 -> 7MSB/1, 7LSB/1
  7LSB/1 -> I/1

== system wlan_phy_rx_pmd/Detect & Decode/Soft Demap/16QAM Soft De-Map/S-R Latch1 ==
  S : Inport
  R : Inport  Port=2
  Constant1 : xbsIndex_r4/Constant  const=1  gui_display_data_type=Fixed-point  arith_type=Boolean  n_bits=1  bin_pt=0  preci_type=Single  exp_width=8  frac_width=24  explicit_period=off  period=1  equ=P=C  opselect=C  inp2=PCIN>>17  opr=+  inp1=P  carry=CIN  dbl_ovrd=off  block_type=constant
      settings: block_version=VER_STRING_GOES_HERE
  Convert1 : xbsIndex_r4/Convert  gui_display_data_type=Boolean  arith_type=Boolean  n_bits=1  bin_pt=0  float_type=Single  exp_bits=8  fraction_bits=24  quantization=Truncate  overflow=Wrap  en=off  latency=0  dbl_ovrd=off  pipeline=off  block_type=convert
  Convert2 : xbsIndex_r4/Convert  gui_display_data_type=Boolean  arith_type=Boolean  n_bits=1  bin_pt=0  float_type=Single  exp_bits=8  fraction_bits=24  quantization=Truncate  overflow=Wrap  en=off  latency=0  dbl_ovrd=off  pipeline=off  block_type=convert
  Register2 : xbsIndex_r4/Register  init=0  rst=on  en=on  dbl_ovrd=off  block_type=register
      settings: xl_area=[1 1 0 0 0 0 0]  block_version=VER_STRING_GOES_HERE
  Q : Outport
  wires:
  S/1 -> Convert2/1
  Register2/1 -> Q/1
  R/1 -> Convert1/1
  Constant1/1 -> Register2/1
  Convert1/1 -> Register2/2
  Convert2/1 -> Register2/3

== system wlan_phy_rx_pmd/Detect & Decode/Soft Demap/64QAM Soft De-Map/Ctrl ==
  Vin : Inport
  Reest : Inport  Port=2
  Constant2 : xbsIndex_r4/Constant  const=2  gui_display_data_type=Fixed-point  arith_type=Unsigned  n_bits=2  bin_pt=0  preci_type=Single  exp_width=8  frac_width=24  explicit_period=off  period=1  equ=P=C  opselect=C  inp2=PCIN>>17  opr=+  inp1=P  carry=CIN  dbl_ovrd=off  block_type=constant
  Counter : xbsIndex_r4/Counter  cnt_type=Count Limited  cnt_to=2  operation=Up  start_count=0  cnt_by_val=1  arith_type=Unsigned  n_bits=2  bin_pt=0  load_pin=off  rst=on  en=on  explicit_period=off  period=1  dbl_ovrd=off  use_behavioral_HDL=off  implementation=Fabric  block_type=counter
  Inverter : xbsIndex_r4/Inverter  en=off  latency=0  dbl_ovrd=off  block_type=inv
      settings: xl_area=[0 0 0 0 0 0 0]  block_version=VER_STRING_GOES_HERE
  Inverter1 : xbsIndex_r4/Inverter  en=off  latency=0  dbl_ovrd=off  block_type=inv
      settings: xl_area=[0 0 0 0 0 0 0]  block_version=VER_STRING_GOES_HERE
  Logical1 : xbsIndex_r4/Logical  logical_function=AND  inputs=2  en=off  latency=0  precision=Full  arith_type=Unsigned  n_bits=16  bin_pt=0  align_bp=on  dbl_ovrd=off  block_type=logical
  Logical2 : xbsIndex_r4/Logical  logical_function=OR  inputs=2  en=off  latency=0  precision=Full  arith_type=Unsigned  n_bits=16  bin_pt=0  align_bp=on  dbl_ovrd=off  block_type=logical
  Logical3 : xbsIndex_r4/Logical  logical_function=OR  inputs=2  en=off  latency=0  precision=Full  arith_type=Unsigned  n_bits=16  bin_pt=0  align_bp=on  dbl_ovrd=off  block_type=logical
  Relational : xbsIndex_r4/Relational  mode=a=b  en=off  latency=0  dbl_ovrd=off  block_type=relational
  S-R Latch1 : Subsystem
  Ready : Outport
  Vout : Outport  Port=2
  Sel : Outport  Port=3
  wires:
  Logical1/1 -> Logical3/1
  Inverter1/1 -> Logical1/1
  Vin/1 -> S-R Latch1/1(S), Inverter1/1
  S-R Latch1/1(Q) -> Vout/1, Inverter/1, Counter/2
  Counter/1 -> Relational/1, Sel/1
  Constant2/1 -> Relational/2
  Relational/1 -> Logical1/2, Logical2/1
  Logical2/1 -> Ready/1
  Inverter/1 -> Logical2/2
  Reest/1 -> Logical3/2, Counter/1
  Logical3/1 -> S-R Latch1/2(R)

== system wlan_phy_rx_pmd/Detect & Decode/Soft Demap/64QAM Soft De-Map/b1/b4 map ==
  IQ : Inport
  Absolute1 : xbsIndex_r4/Absolute  precision=Full  arith_type=Unsigned  n_bits=8  bin_pt=0  quantization=Truncate  overflow=Saturate  en=off  latency=0  dbl_ovrd=off  block_type=abs
  AddSub1 : xbsIndex_r4/AddSub  mode=Subtraction  use_carryin=off  use_carryout=off  en=off  latency=0  precision=User Defined  arith_type="Signed  (2's comp)"  n_bits=8  bin_pt=7  quantization=Truncate  overflow=Saturate  dbl_ovrd=off  use_behavioral_HDL=off  hw_selection=Fabric  pipelined=off  block_type=addsub
  Constant1 : xbsIndex_r4/Constant  const=4/sqrt(42)  gui_display_data_type=Fixed-point  arith_type=Unsigned  n_bits=12  bin_pt=12  preci_type=Single  exp_width=8  frac_width=24  explicit_period=off  period=1  equ=P=C  opselect=C  inp2=PCIN>>17  opr=+  inp1=P  carry=CIN  dbl_ovrd=off  block_type=constant
  b : Outport
  wires:
  Absolute1/1 -> AddSub1/2
  Constant1/1 -> AddSub1/1
  IQ/1 -> Absolute1/1
  AddSub1/1 -> b/1

== system wlan_phy_rx_pmd/Detect & Decode/Soft Demap/64QAM Soft De-Map/b1/b4 map1 ==
  IQ : Inport
  Absolute1 : xbsIndex_r4/Absolute  precision=Full  arith_type=Unsigned  n_bits=8  bin_pt=0  quantization=Truncate  overflow=Saturate  en=off  latency=0  dbl_ovrd=off  block_type=abs
  AddSub1 : xbsIndex_r4/AddSub  mode=Subtraction  use_carryin=off  use_carryout=off  en=off  latency=0  precision=User Defined  arith_type="Signed  (2's comp)"  n_bits=8  bin_pt=7  quantization=Truncate  overflow=Saturate  dbl_ovrd=off  use_behavioral_HDL=off  hw_selection=Fabric  pipelined=off  block_type=addsub
  Constant1 : xbsIndex_r4/Constant  const=4/sqrt(42)  gui_display_data_type=Fixed-point  arith_type=Unsigned  n_bits=12  bin_pt=12  preci_type=Single  exp_width=8  frac_width=24  explicit_period=off  period=1  equ=P=C  opselect=C  inp2=PCIN>>17  opr=+  inp1=P  carry=CIN  dbl_ovrd=off  block_type=constant
  b : Outport
  wires:
  AddSub1/1 -> b/1
  IQ/1 -> Absolute1/1
  Constant1/1 -> AddSub1/1
  Absolute1/1 -> AddSub1/2

== system wlan_phy_rx_pmd/Detect & Decode/Soft Demap/64QAM Soft De-Map/b2/b5 map ==
  IQ : Inport
  Absolute : xbsIndex_r4/Absolute  precision=Full  arith_type=Unsigned  n_bits=8  bin_pt=0  quantization=Truncate  overflow=Saturate  en=off  latency=0  dbl_ovrd=off  block_type=abs
  AddSub : xbsIndex_r4/AddSub  mode=Subtraction  use_carryin=off  use_carryout=off  en=off  latency=0  precision=User Defined  arith_type="Signed  (2's comp)"  n_bits=8  bin_pt=7  quantization=Truncate  overflow=Saturate  dbl_ovrd=off  use_behavioral_HDL=off  hw_selection=Fabric  pipelined=off  block_type=addsub
  AddSub1 : xbsIndex_r4/AddSub  mode=Subtraction  use_carryin=off  use_carryout=off  en=off  latency=0  precision=User Defined  arith_type="Signed  (2's comp)"  n_bits=8  bin_pt=7  quantization=Truncate  overflow=Saturate  dbl_ovrd=off  use_behavioral_HDL=off  hw_selection=Fabric  pipelined=off  block_type=addsub
  Constant : xbsIndex_r4/Constant  const=6/sqrt(42)  gui_display_data_type=Fixed-point  arith_type=Unsigned  n_bits=12  bin_pt=12  preci_type=Single  exp_width=8  frac_width=24  explicit_period=off  period=1  equ=P=C  opselect=C  inp2=PCIN>>17  opr=+  inp1=P  carry=CIN  dbl_ovrd=off  block_type=constant
  Constant1 : xbsIndex_r4/Constant  const=4/sqrt(42)  gui_display_data_type=Fixed-point  arith_type=Unsigned  n_bits=12  bin_pt=12  preci_type=Single  exp_width=8  frac_width=24  explicit_period=off  period=1  equ=P=C  opselect=C  inp2=PCIN>>17  opr=+  inp1=P  carry=CIN  dbl_ovrd=off  block_type=constant
  Constant2 : xbsIndex_r4/Constant  const=2/sqrt(42)  gui_display_data_type=Fixed-point  arith_type=Unsigned  n_bits=12  bin_pt=12  preci_type=Single  exp_width=8  frac_width=24  explicit_period=off  period=1  equ=P=C  opselect=C  inp2=PCIN>>17  opr=+  inp1=P  carry=CIN  dbl_ovrd=off  block_type=constant
  Mux1 : xbsIndex_r4/Mux  inputs=2  en=off  latency=0  precision=Full  arith_type=Unsigned  n_bits=16  bin_pt=14  quantization=Truncate  overflow=Wrap  dbl_ovrd=off  block_type=mux
  Relational : xbsIndex_r4/Relational  mode=a>b  en=off  latency=0  dbl_ovrd=off  block_type=relational
  b : Outport
  wires:
  Mux1/1 -> b/1
  Relational/1 -> Mux1/1
  Constant/1 -> AddSub/1
  IQ/1 -> Absolute/1
  Absolute/1 -> Relational/1, AddSub1/1, AddSub/2
  Constant1/1 -> Relational/2
  AddSub/1 -> Mux1/3
  Constant2/1 -> AddSub1/2
  AddSub1/1 -> Mux1/2

== system wlan_phy_rx_pmd/Detect & Decode/Soft Demap/64QAM Soft De-Map/b2/b5 map1 ==
  IQ : Inport
  Absolute : xbsIndex_r4/Absolute  precision=Full  arith_type=Unsigned  n_bits=8  bin_pt=0  quantization=Truncate  overflow=Saturate  en=off  latency=0  dbl_ovrd=off  block_type=abs
  AddSub : xbsIndex_r4/AddSub  mode=Subtraction  use_carryin=off  use_carryout=off  en=off  latency=0  precision=User Defined  arith_type="Signed  (2's comp)"  n_bits=8  bin_pt=7  quantization=Truncate  overflow=Saturate  dbl_ovrd=off  use_behavioral_HDL=off  hw_selection=Fabric  pipelined=off  block_type=addsub
  AddSub1 : xbsIndex_r4/AddSub  mode=Subtraction  use_carryin=off  use_carryout=off  en=off  latency=0  precision=User Defined  arith_type="Signed  (2's comp)"  n_bits=8  bin_pt=7  quantization=Truncate  overflow=Saturate  dbl_ovrd=off  use_behavioral_HDL=off  hw_selection=Fabric  pipelined=off  block_type=addsub
  Constant : xbsIndex_r4/Constant  const=6/sqrt(42)  gui_display_data_type=Fixed-point  arith_type=Unsigned  n_bits=12  bin_pt=12  preci_type=Single  exp_width=8  frac_width=24  explicit_period=off  period=1  equ=P=C  opselect=C  inp2=PCIN>>17  opr=+  inp1=P  carry=CIN  dbl_ovrd=off  block_type=constant
  Constant1 : xbsIndex_r4/Constant  const=4/sqrt(42)  gui_display_data_type=Fixed-point  arith_type=Unsigned  n_bits=12  bin_pt=12  preci_type=Single  exp_width=8  frac_width=24  explicit_period=off  period=1  equ=P=C  opselect=C  inp2=PCIN>>17  opr=+  inp1=P  carry=CIN  dbl_ovrd=off  block_type=constant
  Constant2 : xbsIndex_r4/Constant  const=2/sqrt(42)  gui_display_data_type=Fixed-point  arith_type=Unsigned  n_bits=12  bin_pt=12  preci_type=Single  exp_width=8  frac_width=24  explicit_period=off  period=1  equ=P=C  opselect=C  inp2=PCIN>>17  opr=+  inp1=P  carry=CIN  dbl_ovrd=off  block_type=constant
  Mux1 : xbsIndex_r4/Mux  inputs=2  en=off  latency=0  precision=Full  arith_type=Unsigned  n_bits=16  bin_pt=14  quantization=Truncate  overflow=Wrap  dbl_ovrd=off  block_type=mux
  Relational : xbsIndex_r4/Relational  mode=a>b  en=off  latency=0  dbl_ovrd=off  block_type=relational
  b : Outport
  wires:
  AddSub1/1 -> Mux1/2
  Constant2/1 -> AddSub1/2
  AddSub/1 -> Mux1/3
  Constant1/1 -> Relational/2
  Absolute/1 -> AddSub/2, AddSub1/1, Relational/1
  IQ/1 -> Absolute/1
  Constant/1 -> AddSub/1
  Relational/1 -> Mux1/1
  Mux1/1 -> b/1

== system wlan_phy_rx_pmd/Detect & Decode/Soft Demap/BPSK Soft De-Map/S-R Latch1 ==
  S : Inport
  R : Inport  Port=2
  Constant1 : xbsIndex_r4/Constant  const=1  gui_display_data_type=Fixed-point  arith_type=Boolean  n_bits=1  bin_pt=0  preci_type=Single  exp_width=8  frac_width=24  explicit_period=off  period=1  equ=P=C  opselect=C  inp2=PCIN>>17  opr=+  inp1=P  carry=CIN  dbl_ovrd=off  block_type=constant
      settings: block_version=VER_STRING_GOES_HERE
  Convert1 : xbsIndex_r4/Convert  gui_display_data_type=Boolean  arith_type=Boolean  n_bits=1  bin_pt=0  float_type=Single  exp_bits=8  fraction_bits=24  quantization=Truncate  overflow=Wrap  en=off  latency=0  dbl_ovrd=off  pipeline=off  block_type=convert
  Convert2 : xbsIndex_r4/Convert  gui_display_data_type=Boolean  arith_type=Boolean  n_bits=1  bin_pt=0  float_type=Single  exp_bits=8  fraction_bits=24  quantization=Truncate  overflow=Wrap  en=off  latency=0  dbl_ovrd=off  pipeline=off  block_type=convert
  Register2 : xbsIndex_r4/Register  init=0  rst=on  en=on  dbl_ovrd=off  block_type=register
      settings: xl_area=[1 1 0 0 0 0 0]  block_version=VER_STRING_GOES_HERE
  Q : Outport
  wires:
  S/1 -> Convert2/1
  Register2/1 -> Q/1
  R/1 -> Convert1/1
  Constant1/1 -> Register2/1
  Convert1/1 -> Register2/2
  Convert2/1 -> Register2/3
